simulink model "filt_oldbase"  (Simulink 8.0) (continued)
== system filt_oldbase/FIR == (continued)
      | a(8) = {'off'};
      | else
      | a(6) = {'on'};
      | a(8) = {'on'};
      | end
      | set_param(gcb, 'MaskEnables', a);
  b60b61 : Subsystem  Tag=xps:sw_reg  AncestorBlock=xps_library/software register  mask{numios=1, io_dir=From Processor, io_delay=0, sample_period=1, name1=reg, bitwidth1=32, arith_type1=Unsigned, bin_pt1=0}
      mask prompts: numios "Num I/Os"; io_dir "I/O direction"; io_delay "I/O delay"; sample_period "Sample period"; name1 "Name"; bitwidth1 "Bitwidth"; arith_type1 "Type"; bin_pt1 "Binary pt"
      mask init:
      | swreg_init(gcb);
      mask Callback numios: t = timer('StartDelay', 0.1); t.TimerFcn = {@swreg_cb, gcb}; start(t);
      mask TypeOptions io_dir: To Processor | From Processor
      mask Callback io_dir:
      | a = get_param(gcb, 'MaskEnables');
      | if strcmp(get_param(gcb, 'io_dir'), 'To Processor'),
      | a(4) = {'off'};
      | else
      | a(4) = {'on'};
      | end
      | set_param(gcb, 'MaskEnables', a);
      mask TypeOptions arith_type1: Unsigned | Signed  (2's comp) | Boolean
      mask Callback arith_type1:
      | a = get_param(gcb, 'MaskEnables');
      | if strcmp(get_param(gcb, 'arith_type1'), 'Boolean'),
      | set_param(gcb, 'bitwidth1', '1');
      | set_param(gcb, 'bin_pt1', '0');
      | a(6) = {'off'};
      | a(8) = {'off'};
      | else
      | a(6) = {'on'};
      | a(8) = {'on'};
      | end
      | set_param(gcb, 'MaskEnables', a);
  b62b63 : Subsystem  Tag=xps:sw_reg  AncestorBlock=xps_library/software register  mask{numios=1, io_dir=From Processor, io_delay=0, sample_period=1, name1=reg, bitwidth1=32, arith_type1=Unsigned, bin_pt1=0}
      mask prompts: numios "Num I/Os"; io_dir "I/O direction"; io_delay "I/O delay"; sample_period "Sample period"; name1 "Name"; bitwidth1 "Bitwidth"; arith_type1 "Type"; bin_pt1 "Binary pt"
      mask init:
      | swreg_init(gcb);
      mask Callback numios: t = timer('StartDelay', 0.1); t.TimerFcn = {@swreg_cb, gcb}; start(t);
      mask TypeOptions io_dir: To Processor | From Processor
      mask Callback io_dir:
      | a = get_param(gcb, 'MaskEnables');
      | if strcmp(get_param(gcb, 'io_dir'), 'To Processor'),
      | a(4) = {'off'};
      | else
      | a(4) = {'on'};
      | end
      | set_param(gcb, 'MaskEnables', a);
      mask TypeOptions arith_type1: Unsigned | Signed  (2's comp) | Boolean
      mask Callback arith_type1:
      | a = get_param(gcb, 'MaskEnables');
      | if strcmp(get_param(gcb, 'arith_type1'), 'Boolean'),
      | set_param(gcb, 'bitwidth1', '1');
      | set_param(gcb, 'bin_pt1', '0');
      | a(6) = {'off'};
      | a(8) = {'off'};
      | else
      | a(6) = {'on'};
      | a(8) = {'on'};
      | end
      | set_param(gcb, 'MaskEnables', a);
  b64b65 : Subsystem  Tag=xps:sw_reg  AncestorBlock=xps_library/software register  mask{numios=1, io_dir=From Processor, io_delay=0, sample_period=1, name1=reg, bitwidth1=32, arith_type1=Unsigned, bin_pt1=0}
      mask prompts: numios "Num I/Os"; io_dir "I/O direction"; io_delay "I/O delay"; sample_period "Sample period"; name1 "Name"; bitwidth1 "Bitwidth"; arith_type1 "Type"; bin_pt1 "Binary pt"
      mask init:
      | swreg_init(gcb);
      mask Callback numios: t = timer('StartDelay', 0.1); t.TimerFcn = {@swreg_cb, gcb}; start(t);
      mask TypeOptions io_dir: To Processor | From Processor
      mask Callback io_dir:
      | a = get_param(gcb, 'MaskEnables');
      | if strcmp(get_param(gcb, 'io_dir'), 'To Processor'),
      | a(4) = {'off'};
      | else
      | a(4) = {'on'};
      | end
      | set_param(gcb, 'MaskEnables', a);
      mask TypeOptions arith_type1: Unsigned | Signed  (2's comp) | Boolean
      mask Callback arith_type1:
      | a = get_param(gcb, 'MaskEnables');
      | if strcmp(get_param(gcb, 'arith_type1'), 'Boolean'),
      | set_param(gcb, 'bitwidth1', '1');
      | set_param(gcb, 'bin_pt1', '0');
      | a(6) = {'off'};
      | a(8) = {'off'};
      | else
      | a(6) = {'on'};
      | a(8) = {'on'};
      | end
      | set_param(gcb, 'MaskEnables', a);
  b66b67 : Subsystem  Tag=xps:sw_reg  AncestorBlock=xps_library/software register  mask{numios=1, io_dir=From Processor, io_delay=0, sample_period=1, name1=reg, bitwidth1=32, arith_type1=Unsigned, bin_pt1=0}
      mask prompts: numios "Num I/Os"; io_dir "I/O direction"; io_delay "I/O delay"; sample_period "Sample period"; name1 "Name"; bitwidth1 "Bitwidth"; arith_type1 "Type"; bin_pt1 "Binary pt"
      mask init:
      | swreg_init(gcb);
      mask Callback numios: t = timer('StartDelay', 0.1); t.TimerFcn = {@swreg_cb, gcb}; start(t);
      mask TypeOptions io_dir: To Processor | From Processor
      mask Callback io_dir:
      | a = get_param(gcb, 'MaskEnables');
      | if strcmp(get_param(gcb, 'io_dir'), 'To Processor'),
      | a(4) = {'off'};
      | else
      | a(4) = {'on'};
      | end
      | set_param(gcb, 'MaskEnables', a);
      mask TypeOptions arith_type1: Unsigned | Signed  (2's comp) | Boolean
      mask Callback arith_type1:
      | a = get_param(gcb, 'MaskEnables');
      | if strcmp(get_param(gcb, 'arith_type1'), 'Boolean'),
      | set_param(gcb, 'bitwidth1', '1');
      | set_param(gcb, 'bin_pt1', '0');
      | a(6) = {'off'};
      | a(8) = {'off'};
      | else
      | a(6) = {'on'};
      | a(8) = {'on'};
      | end
      | set_param(gcb, 'MaskEnables', a);
  b68b69 : Subsystem  Tag=xps:sw_reg  AncestorBlock=xps_library/software register  mask{numios=1, io_dir=From Processor, io_delay=0, sample_period=1, name1=reg, bitwidth1=32, arith_type1=Unsigned, bin_pt1=0}
      mask prompts: numios "Num I/Os"; io_dir "I/O direction"; io_delay "I/O delay"; sample_period "Sample period"; name1 "Name"; bitwidth1 "Bitwidth"; arith_type1 "Type"; bin_pt1 "Binary pt"
      mask init:
      | swreg_init(gcb);
      mask Callback numios: t = timer('StartDelay', 0.1); t.TimerFcn = {@swreg_cb, gcb}; start(t);
      mask TypeOptions io_dir: To Processor | From Processor
      mask Callback io_dir:
      | a = get_param(gcb, 'MaskEnables');
      | if strcmp(get_param(gcb, 'io_dir'), 'To Processor'),
      | a(4) = {'off'};
      | else
      | a(4) = {'on'};
      | end
      | set_param(gcb, 'MaskEnables', a);
      mask TypeOptions arith_type1: Unsigned | Signed  (2's comp) | Boolean
      mask Callback arith_type1:
      | a = get_param(gcb, 'MaskEnables');
      | if strcmp(get_param(gcb, 'arith_type1'), 'Boolean'),
      | set_param(gcb, 'bitwidth1', '1');
      | set_param(gcb, 'bin_pt1', '0');
      | a(6) = {'off'};
      | a(8) = {'off'};
      | else
      | a(6) = {'on'};
      | a(8) = {'on'};
      | end
      | set_param(gcb, 'MaskEnables', a);
  b6b7 : Subsystem  Tag=xps:sw_reg  AncestorBlock=xps_library/software register  mask{numios=1, io_dir=From Processor, io_delay=0, sample_period=1, name1=reg, bitwidth1=32, arith_type1=Unsigned, bin_pt1=0}
      mask prompts: numios "Num I/Os"; io_dir "I/O direction"; io_delay "I/O delay"; sample_period "Sample period"; name1 "Name"; bitwidth1 "Bitwidth"; arith_type1 "Type"; bin_pt1 "Binary pt"
      mask init:
      | swreg_init(gcb);
      mask Callback numios: t = timer('StartDelay', 0.1); t.TimerFcn = {@swreg_cb, gcb}; start(t);
      mask TypeOptions io_dir: To Processor | From Processor
      mask Callback io_dir:
      | a = get_param(gcb, 'MaskEnables');
      | if strcmp(get_param(gcb, 'io_dir'), 'To Processor'),
      | a(4) = {'off'};
      | else
      | a(4) = {'on'};
      | end
      | set_param(gcb, 'MaskEnables', a);
      mask TypeOptions arith_type1: Unsigned | Signed  (2's comp) | Boolean
      mask Callback arith_type1:
      | a = get_param(gcb, 'MaskEnables');
      | if strcmp(get_param(gcb, 'arith_type1'), 'Boolean'),
      | set_param(gcb, 'bitwidth1', '1');
      | set_param(gcb, 'bin_pt1', '0');
      | a(6) = {'off'};
      | a(8) = {'off'};
      | else
      | a(6) = {'on'};
      | a(8) = {'on'};
      | end
      | set_param(gcb, 'MaskEnables', a);
  b70b71 : Subsystem  Tag=xps:sw_reg  AncestorBlock=xps_library/software register  mask{numios=1, io_dir=From Processor, io_delay=0, sample_period=1, name1=reg, bitwidth1=32, arith_type1=Unsigned, bin_pt1=0}
      mask prompts: numios "Num I/Os"; io_dir "I/O direction"; io_delay "I/O delay"; sample_period "Sample period"; name1 "Name"; bitwidth1 "Bitwidth"; arith_type1 "Type"; bin_pt1 "Binary pt"
      mask init:
      | swreg_init(gcb);
      mask Callback numios: t = timer('StartDelay', 0.1); t.TimerFcn = {@swreg_cb, gcb}; start(t);
      mask TypeOptions io_dir: To Processor | From Processor
      mask Callback io_dir:
      | a = get_param(gcb, 'MaskEnables');
      | if strcmp(get_param(gcb, 'io_dir'), 'To Processor'),
      | a(4) = {'off'};
      | else
      | a(4) = {'on'};
      | end
      | set_param(gcb, 'MaskEnables', a);
      mask TypeOptions arith_type1: Unsigned | Signed  (2's comp) | Boolean
      mask Callback arith_type1:
      | a = get_param(gcb, 'MaskEnables');
      | if strcmp(get_param(gcb, 'arith_type1'), 'Boolean'),
      | set_param(gcb, 'bitwidth1', '1');
      | set_param(gcb, 'bin_pt1', '0');
      | a(6) = {'off'};
      | a(8) = {'off'};
      | else
      | a(6) = {'on'};
      | a(8) = {'on'};
      | end
      | set_param(gcb, 'MaskEnables', a);
  b72b73 : Subsystem  Tag=xps:sw_reg  AncestorBlock=xps_library/software register  mask{numios=1, io_dir=From Processor, io_delay=0, sample_period=1, name1=reg, bitwidth1=32, arith_type1=Unsigned, bin_pt1=0}
      mask prompts: numios "Num I/Os"; io_dir "I/O direction"; io_delay "I/O delay"; sample_period "Sample period"; name1 "Name"; bitwidth1 "Bitwidth"; arith_type1 "Type"; bin_pt1 "Binary pt"
      mask init:
      | swreg_init(gcb);
      mask Callback numios: t = timer('StartDelay', 0.1); t.TimerFcn = {@swreg_cb, gcb}; start(t);
      mask TypeOptions io_dir: To Processor | From Processor
      mask Callback io_dir:
      | a = get_param(gcb, 'MaskEnables');
      | if strcmp(get_param(gcb, 'io_dir'), 'To Processor'),
      | a(4) = {'off'};
      | else
      | a(4) = {'on'};
      | end
      | set_param(gcb, 'MaskEnables', a);
      mask TypeOptions arith_type1: Unsigned | Signed  (2's comp) | Boolean
      mask Callback arith_type1:
      | a = get_param(gcb, 'MaskEnables');
      | if strcmp(get_param(gcb, 'arith_type1'), 'Boolean'),
      | set_param(gcb, 'bitwidth1', '1');
      | set_param(gcb, 'bin_pt1', '0');
      | a(6) = {'off'};
      | a(8) = {'off'};
      | else
      | a(6) = {'on'};
      | a(8) = {'on'};
      | end
      | set_param(gcb, 'MaskEnables', a);
  b74b75 : Subsystem  Tag=xps:sw_reg  AncestorBlock=xps_library/software register  mask{numios=1, io_dir=From Processor, io_delay=0, sample_period=1, name1=reg, bitwidth1=32, arith_type1=Unsigned, bin_pt1=0}
      mask prompts: numios "Num I/Os"; io_dir "I/O direction"; io_delay "I/O delay"; sample_period "Sample period"; name1 "Name"; bitwidth1 "Bitwidth"; arith_type1 "Type"; bin_pt1 "Binary pt"
      mask init:
      | swreg_init(gcb);
      mask Callback numios: t = timer('StartDelay', 0.1); t.TimerFcn = {@swreg_cb, gcb}; start(t);
      mask TypeOptions io_dir: To Processor | From Processor
      mask Callback io_dir:
      | a = get_param(gcb, 'MaskEnables');
      | if strcmp(get_param(gcb, 'io_dir'), 'To Processor'),
      | a(4) = {'off'};
      | else
      | a(4) = {'on'};
      | end
      | set_param(gcb, 'MaskEnables', a);
      mask TypeOptions arith_type1: Unsigned | Signed  (2's comp) | Boolean
      mask Callback arith_type1:
      | a = get_param(gcb, 'MaskEnables');
      | if strcmp(get_param(gcb, 'arith_type1'), 'Boolean'),
      | set_param(gcb, 'bitwidth1', '1');
      | set_param(gcb, 'bin_pt1', '0');
      | a(6) = {'off'};
      | a(8) = {'off'};
      | else
      | a(6) = {'on'};
      | a(8) = {'on'};
      | end
      | set_param(gcb, 'MaskEnables', a);
  b76b77 : Subsystem  Tag=xps:sw_reg  AncestorBlock=xps_library/software register  mask{numios=1, io_dir=From Processor, io_delay=0, sample_period=1, name1=reg, bitwidth1=32, arith_type1=Unsigned, bin_pt1=0}
      mask prompts: numios "Num I/Os"; io_dir "I/O direction"; io_delay "I/O delay"; sample_period "Sample period"; name1 "Name"; bitwidth1 "Bitwidth"; arith_type1 "Type"; bin_pt1 "Binary pt"
      mask init:
      | swreg_init(gcb);
      mask Callback numios: t = timer('StartDelay', 0.1); t.TimerFcn = {@swreg_cb, gcb}; start(t);
      mask TypeOptions io_dir: To Processor | From Processor
      mask Callback io_dir:
      | a = get_param(gcb, 'MaskEnables');
      | if strcmp(get_param(gcb, 'io_dir'), 'To Processor'),
      | a(4) = {'off'};
      | else
      | a(4) = {'on'};
      | end
      | set_param(gcb, 'MaskEnables', a);
      mask TypeOptions arith_type1: Unsigned | Signed  (2's comp) | Boolean
      mask Callback arith_type1:
      | a = get_param(gcb, 'MaskEnables');
      | if strcmp(get_param(gcb, 'arith_type1'), 'Boolean'),
      | set_param(gcb, 'bitwidth1', '1');
      | set_param(gcb, 'bin_pt1', '0');
      | a(6) = {'off'};
      | a(8) = {'off'};
      | else
      | a(6) = {'on'};
      | a(8) = {'on'};
      | end
      | set_param(gcb, 'MaskEnables', a);
  b78b79 : Subsystem  Tag=xps:sw_reg  AncestorBlock=xps_library/software register  mask{numios=1, io_dir=From Processor, io_delay=0, sample_period=1, name1=reg, bitwidth1=32, arith_type1=Unsigned, bin_pt1=0}
      mask prompts: numios "Num I/Os"; io_dir "I/O direction"; io_delay "I/O delay"; sample_period "Sample period"; name1 "Name"; bitwidth1 "Bitwidth"; arith_type1 "Type"; bin_pt1 "Binary pt"
      mask init:
      | swreg_init(gcb);
      mask Callback numios: t = timer('StartDelay', 0.1); t.TimerFcn = {@swreg_cb, gcb}; start(t);
      mask TypeOptions io_dir: To Processor | From Processor
      mask Callback io_dir:
      | a = get_param(gcb, 'MaskEnables');
      | if strcmp(get_param(gcb, 'io_dir'), 'To Processor'),
      | a(4) = {'off'};
      | else
      | a(4) = {'on'};
      | end
      | set_param(gcb, 'MaskEnables', a);
      mask TypeOptions arith_type1: Unsigned | Signed  (2's comp) | Boolean
      mask Callback arith_type1:
      | a = get_param(gcb, 'MaskEnables');
      | if strcmp(get_param(gcb, 'arith_type1'), 'Boolean'),
      | set_param(gcb, 'bitwidth1', '1');
      | set_param(gcb, 'bin_pt1', '0');
      | a(6) = {'off'};
      | a(8) = {'off'};
      | else
      | a(6) = {'on'};
      | a(8) = {'on'};
      | end
      | set_param(gcb, 'MaskEnables', a);
  b80b81 : Subsystem  Tag=xps:sw_reg  AncestorBlock=xps_library/software register  mask{numios=1, io_dir=From Processor, io_delay=0, sample_period=1, name1=reg, bitwidth1=32, arith_type1=Unsigned, bin_pt1=0}
      mask prompts: numios "Num I/Os"; io_dir "I/O direction"; io_delay "I/O delay"; sample_period "Sample period"; name1 "Name"; bitwidth1 "Bitwidth"; arith_type1 "Type"; bin_pt1 "Binary pt"
      mask init:
      | swreg_init(gcb);
      mask Callback numios: t = timer('StartDelay', 0.1); t.TimerFcn = {@swreg_cb, gcb}; start(t);
      mask TypeOptions io_dir: To Processor | From Processor
      mask Callback io_dir:
      | a = get_param(gcb, 'MaskEnables');
      | if strcmp(get_param(gcb, 'io_dir'), 'To Processor'),
      | a(4) = {'off'};
      | else
      | a(4) = {'on'};
      | end
      | set_param(gcb, 'MaskEnables', a);
      mask TypeOptions arith_type1: Unsigned | Signed  (2's comp) | Boolean
      mask Callback arith_type1:
      | a = get_param(gcb, 'MaskEnables');
      | if strcmp(get_param(gcb, 'arith_type1'), 'Boolean'),
      | set_param(gcb, 'bitwidth1', '1');
      | set_param(gcb, 'bin_pt1', '0');
      | a(6) = {'off'};
      | a(8) = {'off'};
      | else
      | a(6) = {'on'};
      | a(8) = {'on'};
      | end
      | set_param(gcb, 'MaskEnables', a);
  b82b83 : Subsystem  Tag=xps:sw_reg  AncestorBlock=xps_library/software register  mask{numios=1, io_dir=From Processor, io_delay=0, sample_period=1, name1=reg, bitwidth1=32, arith_type1=Unsigned, bin_pt1=0}
      mask prompts: numios "Num I/Os"; io_dir "I/O direction"; io_delay "I/O delay"; sample_period "Sample period"; name1 "Name"; bitwidth1 "Bitwidth"; arith_type1 "Type"; bin_pt1 "Binary pt"
      mask init:
      | swreg_init(gcb);
      mask Callback numios: t = timer('StartDelay', 0.1); t.TimerFcn = {@swreg_cb, gcb}; start(t);
      mask TypeOptions io_dir: To Processor | From Processor
      mask Callback io_dir:
      | a = get_param(gcb, 'MaskEnables');
      | if strcmp(get_param(gcb, 'io_dir'), 'To Processor'),
      | a(4) = {'off'};
      | else
      | a(4) = {'on'};
      | end
      | set_param(gcb, 'MaskEnables', a);
      mask TypeOptions arith_type1: Unsigned | Signed  (2's comp) | Boolean
      mask Callback arith_type1:
      | a = get_param(gcb, 'MaskEnables');
      | if strcmp(get_param(gcb, 'arith_type1'), 'Boolean'),
      | set_param(gcb, 'bitwidth1', '1');
      | set_param(gcb, 'bin_pt1', '0');
      | a(6) = {'off'};
      | a(8) = {'off'};
      | else
      | a(6) = {'on'};
      | a(8) = {'on'};
      | end
      | set_param(gcb, 'MaskEnables', a);
  b84b85 : Subsystem  Tag=xps:sw_reg  AncestorBlock=xps_library/software register  mask{numios=1, io_dir=From Processor, io_delay=0, sample_period=1, name1=reg, bitwidth1=32, arith_type1=Unsigned, bin_pt1=0}
      mask prompts: numios "Num I/Os"; io_dir "I/O direction"; io_delay "I/O delay"; sample_period "Sample period"; name1 "Name"; bitwidth1 "Bitwidth"; arith_type1 "Type"; bin_pt1 "Binary pt"
      mask init:
      | swreg_init(gcb);
      mask Callback numios: t = timer('StartDelay', 0.1); t.TimerFcn = {@swreg_cb, gcb}; start(t);
      mask TypeOptions io_dir: To Processor | From Processor
      mask Callback io_dir:
      | a = get_param(gcb, 'MaskEnables');
      | if strcmp(get_param(gcb, 'io_dir'), 'To Processor'),
      | a(4) = {'off'};
      | else
      | a(4) = {'on'};
      | end
      | set_param(gcb, 'MaskEnables', a);
      mask TypeOptions arith_type1: Unsigned | Signed  (2's comp) | Boolean
      mask Callback arith_type1:
      | a = get_param(gcb, 'MaskEnables');
      | if strcmp(get_param(gcb, 'arith_type1'), 'Boolean'),
      | set_param(gcb, 'bitwidth1', '1');
      | set_param(gcb, 'bin_pt1', '0');
      | a(6) = {'off'};
      | a(8) = {'off'};
      | else
      | a(6) = {'on'};
      | a(8) = {'on'};
      | end
      | set_param(gcb, 'MaskEnables', a);
  b86b87 : Subsystem  Tag=xps:sw_reg  AncestorBlock=xps_library/software register  mask{numios=1, io_dir=From Processor, io_delay=0, sample_period=1, name1=reg, bitwidth1=32, arith_type1=Unsigned, bin_pt1=0}
      mask prompts: numios "Num I/Os"; io_dir "I/O direction"; io_delay "I/O delay"; sample_period "Sample period"; name1 "Name"; bitwidth1 "Bitwidth"; arith_type1 "Type"; bin_pt1 "Binary pt"
      mask init:
      | swreg_init(gcb);
      mask Callback numios: t = timer('StartDelay', 0.1); t.TimerFcn = {@swreg_cb, gcb}; start(t);
      mask TypeOptions io_dir: To Processor | From Processor
      mask Callback io_dir:
      | a = get_param(gcb, 'MaskEnables');
      | if strcmp(get_param(gcb, 'io_dir'), 'To Processor'),
      | a(4) = {'off'};
      | else
      | a(4) = {'on'};
      | end
      | set_param(gcb, 'MaskEnables', a);
      mask TypeOptions arith_type1: Unsigned | Signed  (2's comp) | Boolean
      mask Callback arith_type1:
      | a = get_param(gcb, 'MaskEnables');
      | if strcmp(get_param(gcb, 'arith_type1'), 'Boolean'),
      | set_param(gcb, 'bitwidth1', '1');
      | set_param(gcb, 'bin_pt1', '0');
      | a(6) = {'off'};
      | a(8) = {'off'};
      | else
      | a(6) = {'on'};
      | a(8) = {'on'};
      | end
      | set_param(gcb, 'MaskEnables', a);
  b88b89 : Subsystem  Tag=xps:sw_reg  AncestorBlock=xps_library/software register  mask{numios=1, io_dir=From Processor, io_delay=0, sample_period=1, name1=reg, bitwidth1=32, arith_type1=Unsigned, bin_pt1=0}
      mask prompts: numios "Num I/Os"; io_dir "I/O direction"; io_delay "I/O delay"; sample_period "Sample period"; name1 "Name"; bitwidth1 "Bitwidth"; arith_type1 "Type"; bin_pt1 "Binary pt"
      mask init:
      | swreg_init(gcb);
      mask Callback numios: t = timer('StartDelay', 0.1); t.TimerFcn = {@swreg_cb, gcb}; start(t);
      mask TypeOptions io_dir: To Processor | From Processor
      mask Callback io_dir:
      | a = get_param(gcb, 'MaskEnables');
      | if strcmp(get_param(gcb, 'io_dir'), 'To Processor'),
      | a(4) = {'off'};
      | else
      | a(4) = {'on'};
      | end
      | set_param(gcb, 'MaskEnables', a);
      mask TypeOptions arith_type1: Unsigned | Signed  (2's comp) | Boolean
      mask Callback arith_type1:
      | a = get_param(gcb, 'MaskEnables');
      | if strcmp(get_param(gcb, 'arith_type1'), 'Boolean'),
      | set_param(gcb, 'bitwidth1', '1');
      | set_param(gcb, 'bin_pt1', '0');
      | a(6) = {'off'};
      | a(8) = {'off'};
      | else
      | a(6) = {'on'};
      | a(8) = {'on'};
      | end
      | set_param(gcb, 'MaskEnables', a);
  b8b9 : Subsystem  Tag=xps:sw_reg  AncestorBlock=xps_library/software register  mask{numios=1, io_dir=From Processor, io_delay=0, sample_period=1, name1=reg, bitwidth1=32, arith_type1=Unsigned, bin_pt1=0}
      mask prompts: numios "Num I/Os"; io_dir "I/O direction"; io_delay "I/O delay"; sample_period "Sample period"; name1 "Name"; bitwidth1 "Bitwidth"; arith_type1 "Type"; bin_pt1 "Binary pt"
      mask init:
      | swreg_init(gcb);
      mask Callback numios: t = timer('StartDelay', 0.1); t.TimerFcn = {@swreg_cb, gcb}; start(t);
      mask TypeOptions io_dir: To Processor | From Processor
      mask Callback io_dir:
      | a = get_param(gcb, 'MaskEnables');
      | if strcmp(get_param(gcb, 'io_dir'), 'To Processor'),
      | a(4) = {'off'};
      | else
      | a(4) = {'on'};
      | end
      | set_param(gcb, 'MaskEnables', a);
      mask TypeOptions arith_type1: Unsigned | Signed  (2's comp) | Boolean
      mask Callback arith_type1:
      | a = get_param(gcb, 'MaskEnables');
      | if strcmp(get_param(gcb, 'arith_type1'), 'Boolean'),
      | set_param(gcb, 'bitwidth1', '1');
      | set_param(gcb, 'bin_pt1', '0');
      | a(6) = {'off'};
      | a(8) = {'off'};
      | else
      | a(6) = {'on'};
      | a(8) = {'on'};
      | end
      | set_param(gcb, 'MaskEnables', a);
  b90b91 : Subsystem  Tag=xps:sw_reg  AncestorBlock=xps_library/software register  mask{numios=1, io_dir=From Processor, io_delay=0, sample_period=1, name1=reg, bitwidth1=32, arith_type1=Unsigned, bin_pt1=0}
      mask prompts: numios "Num I/Os"; io_dir "I/O direction"; io_delay "I/O delay"; sample_period "Sample period"; name1 "Name"; bitwidth1 "Bitwidth"; arith_type1 "Type"; bin_pt1 "Binary pt"
      mask init:
      | swreg_init(gcb);
      mask Callback numios: t = timer('StartDelay', 0.1); t.TimerFcn = {@swreg_cb, gcb}; start(t);
      mask TypeOptions io_dir: To Processor | From Processor
      mask Callback io_dir:
      | a = get_param(gcb, 'MaskEnables');
      | if strcmp(get_param(gcb, 'io_dir'), 'To Processor'),
      | a(4) = {'off'};
      | else
      | a(4) = {'on'};
      | end
      | set_param(gcb, 'MaskEnables', a);
      mask TypeOptions arith_type1: Unsigned | Signed  (2's comp) | Boolean
      mask Callback arith_type1:
      | a = get_param(gcb, 'MaskEnables');
      | if strcmp(get_param(gcb, 'arith_type1'), 'Boolean'),
      | set_param(gcb, 'bitwidth1', '1');
      | set_param(gcb, 'bin_pt1', '0');
      | a(6) = {'off'};
      | a(8) = {'off'};
      | else
      | a(6) = {'on'};
      | a(8) = {'on'};
      | end
      | set_param(gcb, 'MaskEnables', a);
  b92b93 : Subsystem  Tag=xps:sw_reg  AncestorBlock=xps_library/software register  mask{numios=1, io_dir=From Processor, io_delay=0, sample_period=1, name1=reg, bitwidth1=32, arith_type1=Unsigned, bin_pt1=0}
      mask prompts: numios "Num I/Os"; io_dir "I/O direction"; io_delay "I/O delay"; sample_period "Sample period"; name1 "Name"; bitwidth1 "Bitwidth"; arith_type1 "Type"; bin_pt1 "Binary pt"
      mask init:
      | swreg_init(gcb);
      mask Callback numios: t = timer('StartDelay', 0.1); t.TimerFcn = {@swreg_cb, gcb}; start(t);
      mask TypeOptions io_dir: To Processor | From Processor
      mask Callback io_dir:
      | a = get_param(gcb, 'MaskEnables');
      | if strcmp(get_param(gcb, 'io_dir'), 'To Processor'),
      | a(4) = {'off'};
      | else
      | a(4) = {'on'};
      | end
      | set_param(gcb, 'MaskEnables', a);
      mask TypeOptions arith_type1: Unsigned | Signed  (2's comp) | Boolean
      mask Callback arith_type1:
      | a = get_param(gcb, 'MaskEnables');
      | if strcmp(get_param(gcb, 'arith_type1'), 'Boolean'),
      | set_param(gcb, 'bitwidth1', '1');
      | set_param(gcb, 'bin_pt1', '0');
      | a(6) = {'off'};
      | a(8) = {'off'};
      | else
      | a(6) = {'on'};
      | a(8) = {'on'};
      | end
      | set_param(gcb, 'MaskEnables', a);
  b94b95 : Subsystem  Tag=xps:sw_reg  AncestorBlock=xps_library/software register  mask{numios=1, io_dir=From Processor, io_delay=0, sample_period=1, name1=reg, bitwidth1=32, arith_type1=Unsigned, bin_pt1=0}
      mask prompts: numios "Num I/Os"; io_dir "I/O direction"; io_delay "I/O delay"; sample_period "Sample period"; name1 "Name"; bitwidth1 "Bitwidth"; arith_type1 "Type"; bin_pt1 "Binary pt"
      mask init:
      | swreg_init(gcb);
      mask Callback numios: t = timer('StartDelay', 0.1); t.TimerFcn = {@swreg_cb, gcb}; start(t);
      mask TypeOptions io_dir: To Processor | From Processor
      mask Callback io_dir:
      | a = get_param(gcb, 'MaskEnables');
      | if strcmp(get_param(gcb, 'io_dir'), 'To Processor'),
      | a(4) = {'off'};
      | else
      | a(4) = {'on'};
      | end
      | set_param(gcb, 'MaskEnables', a);
      mask TypeOptions arith_type1: Unsigned | Signed  (2's comp) | Boolean
      mask Callback arith_type1:
      | a = get_param(gcb, 'MaskEnables');
      | if strcmp(get_param(gcb, 'arith_type1'), 'Boolean'),
      | set_param(gcb, 'bitwidth1', '1');
      | set_param(gcb, 'bin_pt1', '0');
      | a(6) = {'off'};
      | a(8) = {'off'};
      | else
      | a(6) = {'on'};
      | a(8) = {'on'};
      | end
      | set_param(gcb, 'MaskEnables', a);
  b96b97 : Subsystem  Tag=xps:sw_reg  AncestorBlock=xps_library/software register  mask{numios=1, io_dir=From Processor, io_delay=0, sample_period=1, name1=reg, bitwidth1=32, arith_type1=Unsigned, bin_pt1=0}
      mask prompts: numios "Num I/Os"; io_dir "I/O direction"; io_delay "I/O delay"; sample_period "Sample period"; name1 "Name"; bitwidth1 "Bitwidth"; arith_type1 "Type"; bin_pt1 "Binary pt"
      mask init:
      | swreg_init(gcb);
      mask Callback numios: t = timer('StartDelay', 0.1); t.TimerFcn = {@swreg_cb, gcb}; start(t);
      mask TypeOptions io_dir: To Processor | From Processor
      mask Callback io_dir:
      | a = get_param(gcb, 'MaskEnables');
      | if strcmp(get_param(gcb, 'io_dir'), 'To Processor'),
      | a(4) = {'off'};
      | else
      | a(4) = {'on'};
      | end
      | set_param(gcb, 'MaskEnables', a);
      mask TypeOptions arith_type1: Unsigned | Signed  (2's comp) | Boolean
      mask Callback arith_type1:
      | a = get_param(gcb, 'MaskEnables');
      | if strcmp(get_param(gcb, 'arith_type1'), 'Boolean'),
      | set_param(gcb, 'bitwidth1', '1');
      | set_param(gcb, 'bin_pt1', '0');
      | a(6) = {'off'};
      | a(8) = {'off'};
      | else
      | a(6) = {'on'};
      | a(8) = {'on'};
      | end
      | set_param(gcb, 'MaskEnables', a);
  b98b99 : Subsystem  Tag=xps:sw_reg  AncestorBlock=xps_library/software register  mask{numios=1, io_dir=From Processor, io_delay=0, sample_period=1, name1=reg, bitwidth1=32, arith_type1=Unsigned, bin_pt1=0}
      mask prompts: numios "Num I/Os"; io_dir "I/O direction"; io_delay "I/O delay"; sample_period "Sample period"; name1 "Name"; bitwidth1 "Bitwidth"; arith_type1 "Type"; bin_pt1 "Binary pt"
      mask init:
      | swreg_init(gcb);
      mask Callback numios: t = timer('StartDelay', 0.1); t.TimerFcn = {@swreg_cb, gcb}; start(t);
      mask TypeOptions io_dir: To Processor | From Processor
      mask Callback io_dir:
      | a = get_param(gcb, 'MaskEnables');
      | if strcmp(get_param(gcb, 'io_dir'), 'To Processor'),
      | a(4) = {'off'};
      | else
      | a(4) = {'on'};
      | end
      | set_param(gcb, 'MaskEnables', a);
      mask TypeOptions arith_type1: Unsigned | Signed  (2's comp) | Boolean
      mask Callback arith_type1:
      | a = get_param(gcb, 'MaskEnables');
      | if strcmp(get_param(gcb, 'arith_type1'), 'Boolean'),
      | set_param(gcb, 'bitwidth1', '1');
      | set_param(gcb, 'bin_pt1', '0');
      | a(6) = {'off'};
      | a(8) = {'off'};
      | else
      | a(6) = {'on'};
      | a(8) = {'on'};
      | end
      | set_param(gcb, 'MaskEnables', a);
  load_coeff : Subsystem  Tag=xps:sw_reg  AncestorBlock=xps_library/software register  mask{numios=1, io_dir=From Processor, io_delay=0, sample_period=1, name1=reg, bitwidth1=32, arith_type1=Unsigned, bin_pt1=0}
      mask prompts: numios "Num I/Os"; io_dir "I/O direction"; io_delay "I/O delay"; sample_period "Sample period"; name1 "Name"; bitwidth1 "Bitwidth"; arith_type1 "Type"; bin_pt1 "Binary pt"
      mask init:
      | swreg_init(gcb);
      mask Callback numios: t = timer('StartDelay', 0.1); t.TimerFcn = {@swreg_cb, gcb}; start(t);
      mask TypeOptions io_dir: To Processor | From Processor
      mask Callback io_dir:
      | a = get_param(gcb, 'MaskEnables');
      | if strcmp(get_param(gcb, 'io_dir'), 'To Processor'),
      | a(4) = {'off'};
      | else
      | a(4) = {'on'};
      | end
      | set_param(gcb, 'MaskEnables', a);
      mask TypeOptions arith_type1: Unsigned | Signed  (2's comp) | Boolean
      mask Callback arith_type1:
      | a = get_param(gcb, 'MaskEnables');
      | if strcmp(get_param(gcb, 'arith_type1'), 'Boolean'),
      | set_param(gcb, 'bitwidth1', '1');
      | set_param(gcb, 'bin_pt1', '0');
      | a(6) = {'off'};
      | a(8) = {'off'};
      | else
      | a(6) = {'on'};
      | a(8) = {'on'};
      | end
      | set_param(gcb, 'MaskEnables', a);
  data_out : Outport
  ch_out : Outport  Port=2
  wires:
  Constant26/1 -> b48b49/1(sim_reg)
  Constant25/1 -> b46b47/1(sim_reg)
  Constant24/1 -> b44b45/1(sim_reg)
  Slice77/1 -> Single Port RAM11/2
  Slice76/1 -> Single Port RAM13/2
  Slice75/1 -> Single Port RAM12/2
  Delay12/1 -> Slice77/1
  Delay11/1 -> Slice76/1
  Delay10/1 -> Slice75/1
  Reinterpret49/1 -> FIR_prog/52(coeff50)
  Reinterpret50/1 -> FIR_prog/51(coeff49)
  Reinterpret47/1 -> FIR_prog/50(coeff48)
  Reinterpret48/1 -> FIR_prog/49(coeff47)
  Reinterpret45/1 -> FIR_prog/48(coeff46)
  Reinterpret46/1 -> FIR_prog/47(coeff45)
  [we] -> Single Port RAM11/3
  [we] -> Single Port RAM13/3
  [we] -> Single Port RAM12/3
  [addr] -> Single Port RAM11/1
  [addr] -> Single Port RAM13/1
  [addr] -> Single Port RAM12/1
  Slice74/1 -> Reinterpret49/1
  Slice73/1 -> Reinterpret50/1
  Single Port RAM11/1 -> Slice73/1, Slice74/1
  Slice72/1 -> Reinterpret47/1
  Slice71/1 -> Reinterpret48/1
  Single Port RAM13/1 -> Slice72/1, Slice71/1
  Slice70/1 -> Reinterpret45/1
  Slice69/1 -> Reinterpret46/1
  Single Port RAM12/1 -> Slice69/1, Slice70/1
  b48b49/1(in_reg) -> Delay12/1
  b46b47/1(in_reg) -> Delay11/1
  b44b45/1(in_reg) -> Delay10/1
  Constant23/1 -> b42b43/1(sim_reg)
  Constant22/1 -> b40b41/1(sim_reg)
  Constant21/1 -> b38b39/1(sim_reg)
  Slice68/1 -> Single Port RAM10/2
  Slice67/1 -> Single Port RAM9/2
  Slice66/1 -> Single Port RAM8/2
  Delay9/1 -> Slice68/1
  Delay8/1 -> Slice67/1
  Delay7/1 -> Slice66/1
  Reinterpret43/1 -> FIR_prog/46(coeff44)
  Reinterpret44/1 -> FIR_prog/45(coeff43)
  Reinterpret41/1 -> FIR_prog/44(coeff42)
  Reinterpret42/1 -> FIR_prog/43(coeff41)
  Reinterpret39/1 -> FIR_prog/42(coeff40)
  Reinterpret40/1 -> FIR_prog/41(coeff39)
  [we] -> Single Port RAM10/3
  [we] -> Single Port RAM9/3
  [we] -> Single Port RAM8/3
  [addr] -> Single Port RAM10/1
  [addr] -> Single Port RAM9/1
  [addr] -> Single Port RAM8/1
  Slice65/1 -> Reinterpret43/1
  Slice64/1 -> Reinterpret44/1
  Single Port RAM10/1 -> Slice65/1, Slice64/1
  Slice63/1 -> Reinterpret41/1
  Slice62/1 -> Reinterpret42/1
  Single Port RAM9/1 -> Slice62/1, Slice63/1
  Slice61/1 -> Reinterpret39/1
  Slice60/1 -> Reinterpret40/1
  Single Port RAM8/1 -> Slice61/1, Slice60/1
  b42b43/1(in_reg) -> Delay9/1
  b40b41/1(in_reg) -> Delay8/1
  b38b39/1(in_reg) -> Delay7/1
  Constant20/1 -> b36b37/1(sim_reg)
  Constant19/1 -> b34b35/1(sim_reg)
  Constant18/1 -> b32b33/1(sim_reg)
  Slice59/1 -> Single Port RAM7/2
  Slice58/1 -> Single Port RAM6/2
  Slice57/1 -> Single Port RAM5/2
  Delay6/1 -> Slice59/1
  Delay5/1 -> Slice58/1
  Delay4/1 -> Slice57/1
  Reinterpret37/1 -> FIR_prog/40(coeff38)
  Reinterpret38/1 -> FIR_prog/39(coeff37)
  Reinterpret35/1 -> FIR_prog/38(coeff36)
  Reinterpret36/1 -> FIR_prog/37(coeff35)
  Reinterpret33/1 -> FIR_prog/36(coeff34)
  Reinterpret34/1 -> FIR_prog/35(coeff33)
  [we] -> Single Port RAM7/3
  [we] -> Single Port RAM6/3
  [we] -> Single Port RAM5/3
  [addr] -> Single Port RAM7/1
  [addr] -> Single Port RAM6/1
  [addr] -> Single Port RAM5/1
  Slice56/1 -> Reinterpret37/1
  Slice55/1 -> Reinterpret38/1
  Single Port RAM7/1 -> Slice55/1, Slice56/1
  Slice54/1 -> Reinterpret35/1
  Slice53/1 -> Reinterpret36/1
  Single Port RAM6/1 -> Slice54/1, Slice53/1
  Slice52/1 -> Reinterpret33/1
  Slice51/1 -> Reinterpret34/1
  Single Port RAM5/1 -> Slice51/1, Slice52/1
  b36b37/1(in_reg) -> Delay6/1
  b34b35/1(in_reg) -> Delay5/1
  b32b33/1(in_reg) -> Delay4/1
  Convert/1 -> data_out/1
  Constant17/1 -> load_coeff/1(sim_reg)
  Constant16/1 -> b8b9/1(sim_reg)
  Constant15/1 -> b6b7/1(sim_reg)
  Constant14/1 -> b4b5/1(sim_reg)
  Constant13/1 -> b30b31/1(sim_reg)
  Constant12/1 -> b2b3/1(sim_reg)
  Constant11/1 -> b28b29/1(sim_reg)
  Constant10/1 -> b26b27/1(sim_reg)
  Constant9/1 -> b24b25/1(sim_reg)
  Constant8/1 -> b22b23/1(sim_reg)
  Constant7/1 -> b20b21/1(sim_reg)
  Constant6/1 -> b18b19/1(sim_reg)
  Constant5/1 -> b16b17/1(sim_reg)
  Constant4/1 -> b14b15/1(sim_reg)
  Constant3/1 -> b12b13/1(sim_reg)
  Constant2/1 -> b10b11/1(sim_reg)
  Constant1/1 -> b0b1/1(sim_reg)
  Slice50/1 -> Single Port RAM4/2
  Slice49/1 -> Single Port RAM3/2
  Slice48/1 -> Single Port RAM1/2
  Delay3/1 -> Slice50/1
  Delay2/1 -> Slice49/1
  Delay1/1 -> Slice48/1
  Reinterpret31/1 -> FIR_prog/34(coeff32)
  Reinterpret32/1 -> FIR_prog/33(coeff31)
  Reinterpret29/1 -> FIR_prog/32(coeff30)
  Reinterpret30/1 -> FIR_prog/31(coeff29)
  Reinterpret1/1 -> FIR_prog/30(coeff28)
  Reinterpret28/1 -> FIR_prog/29(coeff27)
  [we] -> Single Port RAM4/3
  [we] -> Single Port RAM3/3
  [we] -> Single Port RAM1/3
  [addr] -> Single Port RAM4/1
  [addr] -> Single Port RAM3/1
  [addr] -> Single Port RAM1/1
  Slice47/1 -> Reinterpret31/1
  Slice46/1 -> Reinterpret32/1
  Single Port RAM4/1 -> Slice47/1, Slice46/1
  Slice45/1 -> Reinterpret29/1
  Slice44/1 -> Reinterpret30/1
  Single Port RAM3/1 -> Slice44/1, Slice45/1
  Slice12/1 -> Reinterpret1/1
  Slice1/1 -> Reinterpret28/1
  Single Port RAM1/1 -> Slice12/1, Slice1/1
  b30b31/1(in_reg) -> Delay3/1
  b28b29/1(in_reg) -> Delay2/1
  b26b27/1(in_reg) -> Delay1/1
  Slice43/1 -> Single Port RAM24/2
  Slice42/1 -> Single Port RAM23/2
  Slice41/1 -> Single Port RAM22/2
  Slice40/1 -> Single Port RAM21/2
  Slice39/1 -> Single Port RAM20/2
  Slice38/1 -> Single Port RAM19/2
  Slice37/1 -> Single Port RAM18/2
  Slice36/1 -> Single Port RAM17/2
  Slice35/1 -> Single Port RAM16/2
  Slice34/1 -> Single Port RAM15/2
  Slice33/1 -> Single Port RAM14/2
  Slice32/1 -> Single Port RAM2/2
  Slice31/1 -> Single Port RAM/2
  Delay44/1 -> Slice43/1
  Delay43/1 -> Slice42/1
  Delay42/1 -> Slice41/1
  Delay41/1 -> Slice40/1
  Delay40/1 -> Slice39/1
  Delay39/1 -> Slice38/1
  Delay38/1 -> Slice37/1
  Delay37/1 -> Slice36/1
  Delay36/1 -> Slice35/1
  Delay35/1 -> Slice34/1
  Delay34/1 -> Slice33/1
  Delay33/1 -> Slice32/1
  Delay32/1 -> Slice31/1
  Delay31/1 -> Slice24/1, Slice23/1
  Delay30/1 -> [we]
  Reinterpret26/1 -> FIR_prog/28(coeff26)
  Reinterpret27/1 -> FIR_prog/27(coeff25)
  Reinterpret24/1 -> FIR_prog/26(coeff24)
  Reinterpret25/1 -> FIR_prog/25(coeff23)
  Reinterpret22/1 -> FIR_prog/24(coeff22)
  Reinterpret23/1 -> FIR_prog/23(coeff21)
  Reinterpret20/1 -> FIR_prog/22(coeff20)
  Reinterpret21/1 -> FIR_prog/21(coeff19)
  Reinterpret18/1 -> FIR_prog/20(coeff18)
  Reinterpret19/1 -> FIR_prog/19(coeff17)
  Reinterpret16/1 -> FIR_prog/18(coeff16)
  Reinterpret17/1 -> FIR_prog/17(coeff15)
  Reinterpret14/1 -> FIR_prog/16(coeff14)
  Reinterpret15/1 -> FIR_prog/15(coeff13)
  Reinterpret12/1 -> FIR_prog/14(coeff12)
  Reinterpret13/1 -> FIR_prog/13(coeff11)
  Reinterpret10/1 -> FIR_prog/12(coeff10)
  Reinterpret11/1 -> FIR_prog/11(coeff9)
  Reinterpret8/1 -> FIR_prog/10(coeff8)
  Reinterpret9/1 -> FIR_prog/9(coeff7)
  Reinterpret6/1 -> FIR_prog/8(coeff6)
  Reinterpret7/1 -> FIR_prog/7(coeff5)
  Reinterpret4/1 -> FIR_prog/6(coeff4)
  Reinterpret5/1 -> FIR_prog/5(coeff3)
  Reinterpret2/1 -> FIR_prog/4(coeff2)
  Reinterpret3/1 -> FIR_prog/3(coeff1)
  Delay23/1 -> Delay29/1
  [we] -> Single Port RAM24/3
  [we] -> Single Port RAM23/3
  [we] -> Single Port RAM22/3
  [we] -> Single Port RAM21/3
  [we] -> Single Port RAM20/3
  [we] -> Single Port RAM19/3
  [we] -> Single Port RAM18/3
  [we] -> Single Port RAM17/3
  [we] -> Single Port RAM16/3
  [we] -> Single Port RAM15/3
  [we] -> Single Port RAM14/3
  [we] -> Single Port RAM2/3
  [we] -> Single Port RAM/3
  [addr] -> Single Port RAM24/1
  [addr] -> Single Port RAM23/1
  [addr] -> Single Port RAM22/1
  [addr] -> Single Port RAM21/1
  [addr] -> Single Port RAM19/1
  [addr] -> Single Port RAM20/1
  [addr] -> Single Port RAM18/1
  [addr] -> Single Port RAM17/1
  [addr] -> Single Port RAM16/1
  [addr] -> Single Port RAM15/1
  [addr] -> Single Port RAM14/1
  [addr] -> Single Port RAM2/1
  [addr] -> Single Port RAM/1
  Mux2/1 -> [addr]
  Slice30/1 -> Reinterpret26/1
  Slice29/1 -> Reinterpret27/1
  Single Port RAM24/1 -> Slice29/1, Slice30/1
  Slice28/1 -> Reinterpret24/1
  Slice27/1 -> Reinterpret25/1
  Single Port RAM23/1 -> Slice28/1, Slice27/1
  Slice26/1 -> Reinterpret22/1
  Slice25/1 -> Reinterpret23/1
  Single Port RAM22/1 -> Slice25/1, Slice26/1
  Slice22/1 -> Reinterpret20/1
  Slice21/1 -> Reinterpret21/1
  Single Port RAM21/1 -> Slice21/1, Slice22/1
  Slice20/1 -> Reinterpret18/1
  Slice19/1 -> Reinterpret19/1
  Single Port RAM20/1 -> Slice20/1, Slice19/1
  Slice18/1 -> Reinterpret16/1
  Slice17/1 -> Reinterpret17/1
  Single Port RAM19/1 -> Slice18/1, Slice17/1
  Slice16/1 -> Reinterpret14/1
  Slice15/1 -> Reinterpret15/1
  Single Port RAM18/1 -> Slice15/1, Slice16/1
  Slice14/1 -> Reinterpret12/1
  Slice13/1 -> Reinterpret13/1
  Single Port RAM17/1 -> Slice13/1, Slice14/1
  Slice11/1 -> Reinterpret10/1
  Slice10/1 -> Reinterpret11/1
  Single Port RAM16/1 -> Slice11/1, Slice10/1
  Slice9/1 -> Reinterpret8/1
  Slice8/1 -> Reinterpret9/1
  Single Port RAM15/1 -> Slice8/1, Slice9/1
  Slice7/1 -> Reinterpret6/1
  Slice6/1 -> Reinterpret7/1
  Single Port RAM14/1 -> Slice7/1, Slice6/1
  Slice5/1 -> Reinterpret4/1
  Slice4/1 -> Reinterpret5/1
  Single Port RAM2/1 -> Slice5/1, Slice4/1
  Slice3/1 -> Reinterpret2/1
  Slice2/1 -> Reinterpret3/1
  Single Port RAM/1 -> Slice3/1, Slice2/1
  b24b25/1(in_reg) -> Delay44/1
  b22b23/1(in_reg) -> Delay43/1
  b20b21/1(in_reg) -> Delay42/1
  b18b19/1(in_reg) -> Delay41/1
  b16b17/1(in_reg) -> Delay40/1
  b14b15/1(in_reg) -> Delay39/1
  b12b13/1(in_reg) -> Delay38/1
  b10b11/1(in_reg) -> Delay37/1
  b8b9/1(in_reg) -> Delay36/1
  b6b7/1(in_reg) -> Delay35/1
  b4b5/1(in_reg) -> Delay34/1
  b2b3/1(in_reg) -> Delay33/1
  b0b1/1(in_reg) -> Delay32/1
  Slice24/1 -> Mux2/3
  Slice23/1 -> Delay30/1, Mux2/1
  load_coeff/1(in_reg) -> Delay31/1
  ch_in/1 -> Delay13/1, Mux2/2
  data_in/1 -> Delay23/1
  FIR_prog/1(sync_out) -> ch_out/1
  Delay13/1 -> Delay14/1
  Delay14/1 -> FIR_prog/1(sync_in)
  Delay29/1 -> FIR_prog/2(data_in)
  Constant44/1 -> b98b99/1(sim_reg)
  Constant43/1 -> b96b97/1(sim_reg)
  Constant42/1 -> b94b95/1(sim_reg)
  Slice150/1 -> Single Port RAM28/2
  Slice149/1 -> Single Port RAM30/2
  Slice148/1 -> Single Port RAM29/2
  Delay18/1 -> Slice150/1
  Delay17/1 -> Slice149/1
  Delay16/1 -> Slice148/1
  [we] -> Single Port RAM28/3
  [we] -> Single Port RAM30/3
  [we] -> Single Port RAM29/3
  [addr] -> Single Port RAM28/1
  [addr] -> Single Port RAM30/1
  [addr] -> Single Port RAM29/1
  Slice147/1 -> Reinterpret94/1
  Slice146/1 -> Reinterpret96/1
  Single Port RAM28/1 -> Slice146/1, Slice147/1
  Slice145/1 -> Reinterpret92/1
  Slice144/1 -> Reinterpret93/1
  Single Port RAM30/1 -> Slice145/1, Slice144/1
  Slice143/1 -> Reinterpret90/1
  Slice141/1 -> Reinterpret91/1
  Single Port RAM29/1 -> Slice141/1, Slice143/1
  b98b99/1(in_reg) -> Delay18/1
  b96b97/1(in_reg) -> Delay17/1
  b94b95/1(in_reg) -> Delay16/1
  Constant41/1 -> b92b93/1(sim_reg)
  Constant40/1 -> b90b91/1(sim_reg)
  Constant39/1 -> b88b89/1(sim_reg)
  Slice140/1 -> Single Port RAM27/2
  Slice139/1 -> Single Port RAM49/2
  Slice138/1 -> Single Port RAM48/2
  Delay56/1 -> Slice140/1
  Delay55/1 -> Slice139/1
  Delay54/1 -> Slice138/1
  [we] -> Single Port RAM27/3
  [we] -> Single Port RAM49/3
  [we] -> Single Port RAM48/3
  [addr] -> Single Port RAM27/1
  [addr] -> Single Port RAM49/1
  [addr] -> Single Port RAM48/1
  Slice137/1 -> Reinterpret88/1
  Slice136/1 -> Reinterpret89/1
  Single Port RAM27/1 -> Slice137/1, Slice136/1
  Slice135/1 -> Reinterpret86/1
  Slice134/1 -> Reinterpret87/1
  Single Port RAM49/1 -> Slice134/1, Slice135/1
  Slice133/1 -> Reinterpret83/1
  Slice132/1 -> Reinterpret85/1
  Single Port RAM48/1 -> Slice133/1, Slice132/1
  b92b93/1(in_reg) -> Delay56/1
  b90b91/1(in_reg) -> Delay55/1
  b88b89/1(in_reg) -> Delay54/1
  Constant38/1 -> b86b87/1(sim_reg)
  Constant36/1 -> b84b85/1(sim_reg)
  Constant35/1 -> b82b83/1(sim_reg)
  Slice130/1 -> Single Port RAM47/2
  Slice129/1 -> Single Port RAM46/2
  Slice128/1 -> Single Port RAM45/2
  Delay53/1 -> Slice130/1
  Delay52/1 -> Slice129/1
  Delay46/1 -> Slice128/1
  [we] -> Single Port RAM47/3
  [we] -> Single Port RAM46/3
  [we] -> Single Port RAM45/3
  [addr] -> Single Port RAM47/1
  [addr] -> Single Port RAM46/1
  [addr] -> Single Port RAM45/1
  Slice127/1 -> Reinterpret81/1
  Slice126/1 -> Reinterpret82/1
  Single Port RAM47/1 -> Slice126/1, Slice127/1
  Slice125/1 -> Reinterpret79/1
  Slice124/1 -> Reinterpret80/1
  Single Port RAM46/1 -> Slice125/1, Slice124/1
  Slice123/1 -> Reinterpret77/1
  Slice122/1 -> Reinterpret78/1
  Single Port RAM45/1 -> Slice122/1, Slice123/1
  b86b87/1(in_reg) -> Delay53/1
  b84b85/1(in_reg) -> Delay52/1
  b82b83/1(in_reg) -> Delay46/1
  Constant34/1 -> b58b59/1(sim_reg)
  Constant33/1 -> b56b57/1(sim_reg)
  Constant32/1 -> b54b55/1(sim_reg)
  Constant31/1 -> b80b81/1(sim_reg)
  Constant30/1 -> b52b53/1(sim_reg)
  Constant29/1 -> b78b79/1(sim_reg)
  Constant28/1 -> b76b77/1(sim_reg)
  Constant51/1 -> b74b75/1(sim_reg)
  Constant50/1 -> b72b73/1(sim_reg)
  Constant49/1 -> b70b71/1(sim_reg)
  Constant48/1 -> b68b69/1(sim_reg)
  Constant47/1 -> b66b67/1(sim_reg)
  Constant46/1 -> b64b65/1(sim_reg)
  Constant45/1 -> b62b63/1(sim_reg)
  Constant37/1 -> b60b61/1(sim_reg)
  Constant27/1 -> b50b51/1(sim_reg)
  Slice121/1 -> Single Port RAM44/2
  Slice119/1 -> Single Port RAM43/2
  Slice118/1 -> Single Port RAM26/2
  Delay20/1 -> Slice121/1
  Delay19/1 -> Slice119/1
  Delay15/1 -> Slice118/1
  [we] -> Single Port RAM44/3
  [we] -> Single Port RAM43/3
  [we] -> Single Port RAM26/3
  [addr] -> Single Port RAM44/1
  [addr] -> Single Port RAM43/1
  [addr] -> Single Port RAM26/1
  Slice117/1 -> Reinterpret75/1
  Slice116/1 -> Reinterpret76/1
  Single Port RAM44/1 -> Slice117/1, Slice116/1
  Slice115/1 -> Reinterpret72/1
  Slice114/1 -> Reinterpret74/1
  Single Port RAM43/1 -> Slice114/1, Slice115/1
  Slice81/1 -> Reinterpret51/1
  Slice78/1 -> Reinterpret71/1
  Single Port RAM26/1 -> Slice81/1, Slice78/1
  b80b81/1(in_reg) -> Delay20/1
  b78b79/1(in_reg) -> Delay19/1
  b76b77/1(in_reg) -> Delay15/1
  Slice113/1 -> Single Port RAM42/2
  Slice112/1 -> Single Port RAM41/2
  Slice111/1 -> Single Port RAM40/2
  Slice110/1 -> Single Port RAM39/2
  Slice108/1 -> Single Port RAM38/2
  Slice107/1 -> Single Port RAM36/2
  Slice106/1 -> Single Port RAM35/2
  Slice105/1 -> Single Port RAM34/2
  Slice104/1 -> Single Port RAM33/2
  Slice103/1 -> Single Port RAM32/2
  Slice102/1 -> Single Port RAM31/2
  Slice101/1 -> Single Port RAM37/2
  Slice100/1 -> Single Port RAM25/2
  Delay51/1 -> Slice113/1
  Delay50/1 -> Slice112/1
  Delay49/1 -> Slice111/1
  Delay48/1 -> Slice110/1
  Delay47/1 -> Slice108/1
  Delay45/1 -> Slice107/1
  Delay28/1 -> Slice106/1
  Delay27/1 -> Slice105/1
  Delay26/1 -> Slice104/1
  Delay25/1 -> Slice103/1
  Delay24/1 -> Slice102/1
  Delay22/1 -> Slice101/1
  Delay21/1 -> Slice100/1
  [we] -> Single Port RAM42/3
  [we] -> Single Port RAM41/3
  [we] -> Single Port RAM40/3
  [we] -> Single Port RAM39/3
  [we] -> Single Port RAM38/3
  [we] -> Single Port RAM36/3
  [we] -> Single Port RAM35/3
  [we] -> Single Port RAM34/3
  [we] -> Single Port RAM33/3
  [we] -> Single Port RAM32/3
  [we] -> Single Port RAM31/3
  [we] -> Single Port RAM37/3
  [we] -> Single Port RAM25/3
  [addr] -> Single Port RAM42/1
  [addr] -> Single Port RAM41/1
  [addr] -> Single Port RAM40/1
  [addr] -> Single Port RAM39/1
  [addr] -> Single Port RAM36/1
  [addr] -> Single Port RAM38/1
  [addr] -> Single Port RAM35/1
  [addr] -> Single Port RAM34/1
  [addr] -> Single Port RAM33/1
  [addr] -> Single Port RAM32/1
  [addr] -> Single Port RAM31/1
  [addr] -> Single Port RAM37/1
  [addr] -> Single Port RAM25/1
  Slice99/1 -> Reinterpret69/1
  Slice97/1 -> Reinterpret70/1
  Single Port RAM42/1 -> Slice97/1, Slice99/1
  Slice96/1 -> Reinterpret67/1
  Slice95/1 -> Reinterpret68/1
  Single Port RAM41/1 -> Slice96/1, Slice95/1
  Slice94/1 -> Reinterpret65/1
  Slice93/1 -> Reinterpret66/1
  Single Port RAM40/1 -> Slice93/1, Slice94/1
  Slice92/1 -> Reinterpret63/1
  Slice91/1 -> Reinterpret64/1
  Single Port RAM39/1 -> Slice91/1, Slice92/1
  Slice90/1 -> Reinterpret60/1
  Slice88/1 -> Reinterpret61/1
  Single Port RAM38/1 -> Slice90/1, Slice88/1
  Slice87/1 -> Reinterpret58/1
  Slice86/1 -> Reinterpret59/1
  Single Port RAM36/1 -> Slice87/1, Slice86/1
  Slice85/1 -> Reinterpret56/1
  Slice84/1 -> Reinterpret57/1
  Single Port RAM35/1 -> Slice84/1, Slice85/1
  Slice83/1 -> Reinterpret54/1
  Slice82/1 -> Reinterpret55/1
  Single Port RAM34/1 -> Slice82/1, Slice83/1
  Slice80/1 -> Reinterpret52/1
  Slice79/1 -> Reinterpret53/1
  Single Port RAM33/1 -> Slice80/1, Slice79/1
  Slice152/1 -> Reinterpret99/1
  Slice151/1 -> Reinterpret100/1
  Single Port RAM32/1 -> Slice151/1, Slice152/1
  Slice142/1 -> Reinterpret97/1
  Slice131/1 -> Reinterpret98/1
  Single Port RAM31/1 -> Slice142/1, Slice131/1
  Slice120/1 -> Reinterpret84/1
  Slice109/1 -> Reinterpret95/1
  Single Port RAM37/1 -> Slice120/1, Slice109/1
  Slice98/1 -> Reinterpret62/1
  Slice89/1 -> Reinterpret73/1
  Single Port RAM25/1 -> Slice98/1, Slice89/1
  b74b75/1(in_reg) -> Delay51/1
  b72b73/1(in_reg) -> Delay50/1
  b70b71/1(in_reg) -> Delay49/1
  b68b69/1(in_reg) -> Delay48/1
  b66b67/1(in_reg) -> Delay47/1
  b64b65/1(in_reg) -> Delay45/1
  b62b63/1(in_reg) -> Delay28/1
  b60b61/1(in_reg) -> Delay27/1
  b58b59/1(in_reg) -> Delay26/1
  b56b57/1(in_reg) -> Delay25/1
  b54b55/1(in_reg) -> Delay24/1
  b52b53/1(in_reg) -> Delay22/1
  b50b51/1(in_reg) -> Delay21/1
  Reinterpret73/1 -> FIR_prog/53(coeff51)
  Reinterpret62/1 -> FIR_prog/54(coeff52)
  Reinterpret95/1 -> FIR_prog/55(coeff53)
  Reinterpret84/1 -> FIR_prog/56(coeff54)
  Reinterpret98/1 -> FIR_prog/57(coeff55)
  Reinterpret97/1 -> FIR_prog/58(coeff56)
  Reinterpret100/1 -> FIR_prog/59(coeff57)
  Reinterpret99/1 -> FIR_prog/60(coeff58)
  Reinterpret53/1 -> FIR_prog/61(coeff59)
  Reinterpret52/1 -> FIR_prog/62(coeff60)
  Reinterpret55/1 -> FIR_prog/63(coeff61)
  Reinterpret54/1 -> FIR_prog/64(coeff62)
  Reinterpret57/1 -> FIR_prog/65(coeff63)
  Reinterpret56/1 -> FIR_prog/66(coeff64)
  Reinterpret59/1 -> FIR_prog/67(coeff65)
  Reinterpret58/1 -> FIR_prog/68(coeff66)
  Reinterpret61/1 -> FIR_prog/69(coeff67)
  Reinterpret60/1 -> FIR_prog/70(coeff68)
  Reinterpret64/1 -> FIR_prog/71(coeff69)
  Reinterpret63/1 -> FIR_prog/72(coeff70)
  Reinterpret66/1 -> FIR_prog/73(coeff71)
  Reinterpret65/1 -> FIR_prog/74(coeff72)
  Reinterpret68/1 -> FIR_prog/75(coeff73)
  Reinterpret67/1 -> FIR_prog/76(coeff74)
  Reinterpret70/1 -> FIR_prog/77(coeff75)
  Reinterpret69/1 -> FIR_prog/78(coeff76)
  Reinterpret71/1 -> FIR_prog/79(coeff77)
  Reinterpret51/1 -> FIR_prog/80(coeff78)
  Reinterpret74/1 -> FIR_prog/81(coeff79)
  Reinterpret72/1 -> FIR_prog/82(coeff80)
  Reinterpret76/1 -> FIR_prog/83(coeff81)
  Reinterpret75/1 -> FIR_prog/84(coeff82)
  Reinterpret78/1 -> FIR_prog/85(coeff83)
  Reinterpret77/1 -> FIR_prog/86(coeff84)
  Reinterpret80/1 -> FIR_prog/87(coeff85)
  Reinterpret79/1 -> FIR_prog/88(coeff86)
  Reinterpret82/1 -> FIR_prog/89(coeff87)
  Reinterpret81/1 -> FIR_prog/90(coeff88)
  Reinterpret85/1 -> FIR_prog/91(coeff89)
  Reinterpret83/1 -> FIR_prog/92(coeff90)
  Reinterpret87/1 -> FIR_prog/93(coeff91)
  Reinterpret86/1 -> FIR_prog/94(coeff92)
  Reinterpret89/1 -> FIR_prog/95(coeff93)
  Reinterpret88/1 -> FIR_prog/96(coeff94)
  Reinterpret91/1 -> FIR_prog/97(coeff95)
  Reinterpret90/1 -> FIR_prog/98(coeff96)
  Reinterpret93/1 -> FIR_prog/99(coeff97)
  Reinterpret92/1 -> FIR_prog/100(coeff98)
  Reinterpret96/1 -> FIR_prog/101(coeff99)
  Reinterpret94/1 -> FIR_prog/102(coeff100)
  FIR_prog/2(data_out) -> Convert/1
